simulink model "ClimaCenCfgMgr"  (R2022b) (continued)
== system ClimaCenCfgMgr == (continued)
  CarCfgForClimaCtrl_CarCfgForRemEngStrtPreCdn/1 -> ClimaCenCfgMgr_Runnable_sys/36(CarCfgForClimaCtrl_CarCfgForRemEngStrtPreCdn_read)
  CarCfgForClimaCtrl_CarCfgForRoofHatch/1 -> ClimaCenCfgMgr_Runnable_sys/37(CarCfgForClimaCtrl_CarCfgForRoofHatch_read)
  CarCfgForClimaCtrl_CarCfgForSeatHeatgCal/1 -> ClimaCenCfgMgr_Runnable_sys/38(CarCfgForClimaCtrl_CarCfgForSeatHeatgCal_read)
  CarCfgForClimaCtrl_CarCfgForSeatHeatgForRowFirst/1 -> ClimaCenCfgMgr_Runnable_sys/39(CarCfgForClimaCtrl_CarCfgForSeatHeatgForRowFirst_read)
  CarCfgForClimaCtrl_CarCfgForSeatHeatgForRowSec/1 -> ClimaCenCfgMgr_Runnable_sys/40(CarCfgForClimaCtrl_CarCfgForSeatHeatgForRowSec_read)
  CarCfgForClimaCtrl_CarCfgForSeatVentnForRowFirst/1 -> ClimaCenCfgMgr_Runnable_sys/41(CarCfgForClimaCtrl_CarCfgForSeatVentnForRowFirst_read)
  CarCfgForClimaCtrl_CarCfgForSeatVentnForRowSec/1 -> ClimaCenCfgMgr_Runnable_sys/42(CarCfgForClimaCtrl_CarCfgForSeatVentnForRowSec_read)
  CarCfgForClimaCtrl_CarCfgForSecRowVentnCenter/1 -> ClimaCenCfgMgr_Runnable_sys/43(CarCfgForClimaCtrl_CarCfgForSecRowVentnCenter_read)
  CarCfgForClimaCtrl_CarCfgForSecRowVentnSide/1 -> ClimaCenCfgMgr_Runnable_sys/44(CarCfgForClimaCtrl_CarCfgForSecRowVentnSide_read)
  CarCfgForClimaCtrl_CarCfgForSmallFlowCyc/1 -> ClimaCenCfgMgr_Runnable_sys/45(CarCfgForClimaCtrl_CarCfgForSmallFlowCyc_read)
  CarCfgForClimaCtrl_CarCfgForSmkSnsr/1 -> ClimaCenCfgMgr_Runnable_sys/46(CarCfgForClimaCtrl_CarCfgForSmkSnsr_read)
  CarCfgForClimaCtrl_CarCfgForSnsrForCmptmtT/1 -> ClimaCenCfgMgr_Runnable_sys/47(CarCfgForClimaCtrl_CarCfgForSnsrForCmptmtT_read)
  CarCfgForClimaCtrl_CarCfgForSnsrHumPrsnt/1 -> ClimaCenCfgMgr_Runnable_sys/48(CarCfgForClimaCtrl_CarCfgForSnsrHumPrsnt_read)
  CarCfgForClimaCtrl_CarCfgForStartDevice/1 -> ClimaCenCfgMgr_Runnable_sys/49(CarCfgForClimaCtrl_CarCfgForStartDevice_read)
  CarCfgForClimaCtrl_CarCfgForSteerWhlHeatg/1 -> ClimaCenCfgMgr_Runnable_sys/50(CarCfgForClimaCtrl_CarCfgForSteerWhlHeatg_read)
  CarCfgForClimaCtrl_CarCfgForSteerWhlPosn/1 -> ClimaCenCfgMgr_Runnable_sys/51(CarCfgForClimaCtrl_CarCfgForSteerWhlPosn_read)
  CarCfgForClimaCtrl_CarCfgForSunSnsr/1 -> ClimaCenCfgMgr_Runnable_sys/52(CarCfgForClimaCtrl_CarCfgForSunSnsr_read)
  CarCfgForClimaCtrl_CarCfgForTelmNodTyp/1 -> ClimaCenCfgMgr_Runnable_sys/53(CarCfgForClimaCtrl_CarCfgForTelmNodTyp_read)
  CarCfgForClimaCtrl_CarCfgForThermMngt/1 -> ClimaCenCfgMgr_Runnable_sys/54(CarCfgForClimaCtrl_CarCfgForThermMngt_read)
  CarCfgForClimaCtrl_CarCfgForVehTyp/1 -> ClimaCenCfgMgr_Runnable_sys/55(CarCfgForClimaCtrl_CarCfgForVehTyp_read)
  CarCfgForClimaCtrl_CarCfgForVgaMotorExistSts/1 -> ClimaCenCfgMgr_Runnable_sys/56(CarCfgForClimaCtrl_CarCfgForVgaMotorExistSts_read)
  CarCfgForClimaCtrl_CarCfgForWindHeatd/1 -> ClimaCenCfgMgr_Runnable_sys/57(CarCfgForClimaCtrl_CarCfgForWindHeatd_read)
  CooltVlvOutpTyp_CooltVlvOutpTyp/1 -> ClimaCenCfgMgr_Runnable_sys/58(CooltVlvOutpTyp_CooltVlvOutpTyp_read)
  HvacCfgForClimaCtrl_HvacCfgForHvacFrontBlowerType/1 -> ClimaCenCfgMgr_Runnable_sys/59(HvacCfgForClimaCtrl_HvacCfgForHvacFrontBlowerType_read)
  HvacCfgForClimaCtrl_HvacCfgForHvacMiscExistSts/1 -> ClimaCenCfgMgr_Runnable_sys/60(HvacCfgForClimaCtrl_HvacCfgForHvacMiscExistSts_read)
  HvacCfgForClimaCtrl_HvacCfgForHvacMotorExistSts/1 -> ClimaCenCfgMgr_Runnable_sys/61(HvacCfgForClimaCtrl_HvacCfgForHvacMotorExistSts_read)
  HvacCfgForClimaCtrl_HvacCfgForHvacSensorExistSts/1 -> ClimaCenCfgMgr_Runnable_sys/62(HvacCfgForClimaCtrl_HvacCfgForHvacSensorExistSts_read)
  CarCfgForClimaCtrl_CarCfgFor3RDROWSEATHEAT/1 -> ClimaCenCfgMgr_Runnable_sys/63(CarCfgForClimaCtrl_CarCfgFor3RDROWSEATHEAT_read)
  CarCfgForClimaCtrl_CarCfgFor3RDROWSEATVENTILATED/1 -> ClimaCenCfgMgr_Runnable_sys/64(CarCfgForClimaCtrl_CarCfgFor3RDROWSEATVENTILATED_read)
  CarCfgForClimaCtrl_CarCfgForBRAND/1 -> ClimaCenCfgMgr_Runnable_sys/65(CarCfgForClimaCtrl_CarCfgForBRAND_read)
  CarCfgForClimaCtrl_CarCfgForCabinOxgenSupply/1 -> ClimaCenCfgMgr_Runnable_sys/66(CarCfgForClimaCtrl_CarCfgForCabinOxgenSupply_read)
  CarCfgForClimaCtrl_CarCfgForElectricLiftingModule/1 -> ClimaCenCfgMgr_Runnable_sys/67(CarCfgForClimaCtrl_CarCfgForElectricLiftingModule_read)
  CarCfgForClimaCtrl_CarCfgForFloatingDriving/1 -> ClimaCenCfgMgr_Runnable_sys/68(CarCfgForClimaCtrl_CarCfgForFloatingDriving_read)
  CarCfgForClimaCtrl_CarCfgForInteriorViewCamera/1 -> ClimaCenCfgMgr_Runnable_sys/69(CarCfgForClimaCtrl_CarCfgForInteriorViewCamera_read)
  CarCfgForClimaCtrl_CarCfgForLifeDetection/1 -> ClimaCenCfgMgr_Runnable_sys/70(CarCfgForClimaCtrl_CarCfgForLifeDetection_read)
  CarCfgForClimaCtrl_CarCfgForMARKET/1 -> ClimaCenCfgMgr_Runnable_sys/71(CarCfgForClimaCtrl_CarCfgForMARKET_read)
  CarCfgForClimaCtrl_CarCfgForPowerSeats/1 -> ClimaCenCfgMgr_Runnable_sys/72(CarCfgForClimaCtrl_CarCfgForPowerSeats_read)
  CarCfgForClimaCtrl_CarCfgForPTCInFrontHVAC/1 -> ClimaCenCfgMgr_Runnable_sys/73(CarCfgForClimaCtrl_CarCfgForPTCInFrontHVAC_read)
  CarCfgForClimaCtrl_CarCfgForTEMPEREDSTORAGEBOX/1 -> ClimaCenCfgMgr_Runnable_sys/75(CarCfgForClimaCtrl_CarCfgForTEMPEREDSTORAGEBOX_read)
  CarCfgForClimaCtrl_CarCfgForVentLiftMotorExiSts/1 -> ClimaCenCfgMgr_Runnable_sys/76(CarCfgForClimaCtrl_CarCfgForVentLiftMotorExiSts_read)
  CarCfgForClimaCtrl_CarCfgForWadingAssistSystem/1 -> ClimaCenCfgMgr_Runnable_sys/77(CarCfgForClimaCtrl_CarCfgForWadingAssistSystem_read)
  CarCfgForClimaCtrl_CarCfgWindFollowHumanMov/1 -> ClimaCenCfgMgr_Runnable_sys/78(CarCfgForClimaCtrl_CarCfgWindFollowHumanMov_read)
  CenCfgMgrForCompile_CarCfgForAirClnrAdv/1 -> ClimaCenCfgMgr_Runnable_sys/79(CenCfgMgrForCompile_CarCfgForAirClnrAdv_read)
  CenCfgMgrForCompile_CarCfgForAutDemst/1 -> ClimaCenCfgMgr_Runnable_sys/80(CenCfgMgrForCompile_CarCfgForAutDemst_read)
  CenCfgMgrForCompile_CarCfgForCmprAcTyp3/1 -> ClimaCenCfgMgr_Runnable_sys/81(CenCfgMgrForCompile_CarCfgForCmprAcTyp3_read)
  CenCfgMgrForCompile_CarCfgForHeatrTypGly/1 -> ClimaCenCfgMgr_Runnable_sys/82(CenCfgMgrForCompile_CarCfgForHeatrTypGly_read)
  CenCfgMgrForCompile_CarCfgForSteerWhlHeatg/1 -> ClimaCenCfgMgr_Runnable_sys/83(CenCfgMgrForCompile_CarCfgForSteerWhlHeatg_read)
  HvacCfgForClimaCtrl_HvacCfgForAQSTypes/1 -> ClimaCenCfgMgr_Runnable_sys/84(HvacCfgForClimaCtrl_HvacCfgForAQSTypes_read)
  HvacCfgForClimaCtrl_HvacCfgForHvacLowSideRETXVESts/1 -> ClimaCenCfgMgr_Runnable_sys/85(HvacCfgForClimaCtrl_HvacCfgForHvacLowSideRETXVESts_read)
  HvacCfgForClimaCtrl_HvacCfgForHvacReBlowerType/1 -> ClimaCenCfgMgr_Runnable_sys/86(HvacCfgForClimaCtrl_HvacCfgForHvacReBlowerType_read)
  HvacCfgForClimaCtrl_HvacCfgForHvacReVentPstn/1 -> ClimaCenCfgMgr_Runnable_sys/87(HvacCfgForClimaCtrl_HvacCfgForHvacReVentPstn_read)
  HvacCfgForClimaCtrl_HvacCfgForHvacSnsrRCmptmtESts/1 -> ClimaCenCfgMgr_Runnable_sys/88(HvacCfgForClimaCtrl_HvacCfgForHvacSnsrRCmptmtESts_read)
  HvacCfgForClimaCtrl_HvacCfgForHvacSnsrReHexESts/1 -> ClimaCenCfgMgr_Runnable_sys/89(HvacCfgForClimaCtrl_HvacCfgForHvacSnsrReHexESts_read)
  HvacCfgReqVersionReplace_HvacCfgReqVersionReplace/1 -> ClimaCenCfgMgr_Runnable_sys/90(HvacCfgReqVersionReplace_HvacCfgReqVersionReplace_read)
  ClimaCenCfgMgr_Runnable_sys/1(ClimaCfgFor3RDROWSEATHEAT_ClimaCfgFor3RDROWSEATHEAT_write) -> ClimaCfgFor3RDROWSEATHEAT_ClimaCfgFor3RDROWSEATHEAT/1
  ClimaCenCfgMgr_Runnable_sys/2(ClimaCfgFor3RDROWSEATVENTILATED_ClimaCfgFor3RDROWSEATVENTILATED_write) -> ClimaCfgFor3RDROWSEATVENTILATED_ClimaCfgFor3RDROWSEATVENTILATED/1
  ClimaCenCfgMgr_Runnable_sys/3(ClimaCfgForAAC_ClimaCfgForAAC_write) -> ClimaCfgForAAC_ClimaCfgForAAC/1
  ClimaCenCfgMgr_Runnable_sys/4(ClimaCfgForAQSTypes_ClimaCfgForAQSTypes_write) -> ClimaCfgForAQSTypes_ClimaCfgForAQSTypes/1
  ClimaCenCfgMgr_Runnable_sys/5(ClimaCfgForAutDemst_ClimaCfgForAutDemst_write) -> ClimaCfgForAutDemst_ClimaCfgForAutDemst/1
  ClimaCenCfgMgr_Runnable_sys/6(ClimaCfgForBRAND_ClimaCfgForBRAND_write) -> ClimaCfgForBRAND_ClimaCfgForBRAND/1
  ClimaCenCfgMgr_Runnable_sys/7(ClimaCfgForCabinOxgenSupply_ClimaCfgForCabinOxgenSupply_write) -> ClimaCfgForCabinOxgenSupply_ClimaCfgForCabinOxgenSupply/1
  ClimaCenCfgMgr_Runnable_sys/8(ClimaCfgForDrvrSeatVentWthSmf2_ClimaCfgForDrvrSeatVentWthSmf2_write) -> ClimaCfgForDrvrSeatVentWthSmf2_ClimaCfgForDrvrSeatVentWthSmf2/1
  ClimaCenCfgMgr_Runnable_sys/9(ClimaCfgForElectricLiftingModule_ClimaCfgForElectricLiftingModule_write) -> ClimaCfgForElectricLiftingModule_ClimaCfgForElectricLiftingModule/1
  ClimaCenCfgMgr_Runnable_sys/10(ClimaCfgForErev1_ClimaCfgForErev1_write) -> ClimaCfgForErev1_ClimaCfgForErev1/1
  ClimaCenCfgMgr_Runnable_sys/11(ClimaCfgForFLOATINGDRVING_ClimaCfgForFLOATINGDRVING_write) -> ClimaCfgForFLOATINGDRVING_ClimaCfgForFLOATINGDRVING/1
  ClimaCenCfgMgr_Runnable_sys/12(ClimaCfgForHvacLowSideRETXVESts_ClimaCfgForHvacLowSideRETXVESts_write) -> ClimaCfgForHvacLowSideRETXVESts_ClimaCfgForHvacLowSideRETXVESts/1
  ClimaCenCfgMgr_Runnable_sys/13(ClimaCfgForHvacReBlowerType_ClimaCfgForHvacReBlowerType_write) -> ClimaCfgForHvacReBlowerType_ClimaCfgForHvacReBlowerType/1
  ClimaCenCfgMgr_Runnable_sys/14(ClimaCfgForHvacReTmpZn_ClimaCfgForHvacReTmpZn_write) -> ClimaCfgForHvacReTmpZn_ClimaCfgForHvacReTmpZn/1
  ClimaCenCfgMgr_Runnable_sys/15(ClimaCfgForHvacReVentPstn_ClimaCfgForRowSecAtFlrLeRe_write) -> ClimaCfgForHvacReVentPstn_ClimaCfgForRowSecAtFlrLeRe/1
  ClimaCenCfgMgr_Runnable_sys/16(ClimaCfgForHvacReVentPstn_ClimaCfgForRowSecAtFlrRiRe_write) -> ClimaCfgForHvacReVentPstn_ClimaCfgForRowSecAtFlrRiRe/1
  ClimaCenCfgMgr_Runnable_sys/17(ClimaCfgForHvacReVentPstn_ClimaCfgForRowSecAtVentSideTopLe_write) -> ClimaCfgForHvacReVentPstn_ClimaCfgForRowSecAtVentSideTopLe/1
  ClimaCenCfgMgr_Runnable_sys/18(ClimaCfgForHvacReVentPstn_ClimaCfgForRowSecAtVentSideTopRi_write) -> ClimaCfgForHvacReVentPstn_ClimaCfgForRowSecAtVentSideTopRi/1
  ClimaCenCfgMgr_Runnable_sys/19(ClimaCfgForHvacReVentPstn_ClimaCfgForRowThrdAtFlrLe_write) -> ClimaCfgForHvacReVentPstn_ClimaCfgForRowThrdAtFlrLe/1
  ClimaCenCfgMgr_Runnable_sys/20(ClimaCfgForHvacReVentPstn_ClimaCfgForRowThrdAtFlrRi_write) -> ClimaCfgForHvacReVentPstn_ClimaCfgForRowThrdAtFlrRi/1
  ClimaCenCfgMgr_Runnable_sys/21(ClimaCfgForHvacReVentPstn_ClimaCfgForRowThrdAtVentSideTopLe_write) -> ClimaCfgForHvacReVentPstn_ClimaCfgForRowThrdAtVentSideTopLe/1
  ClimaCenCfgMgr_Runnable_sys/22(ClimaCfgForHvacReVentPstn_ClimaCfgForRowThrdAtVentSideTopRi_write) -> ClimaCfgForHvacReVentPstn_ClimaCfgForRowThrdAtVentSideTopRi/1
  ClimaCenCfgMgr_Runnable_sys/23(ClimaCfgForHvacSnsrRCmptmtESts_ClimaCfgForHvacSnsrRCmptmtESts_write) -> ClimaCfgForHvacSnsrRCmptmtESts_ClimaCfgForHvacSnsrRCmptmtESts/1
  ClimaCenCfgMgr_Runnable_sys/24(ClimaCfgForHvacSnsrReHexESts_ClimaCfgForHvacSnsrReHexESts_write) -> ClimaCfgForHvacSnsrReHexESts_ClimaCfgForHvacSnsrReHexESts/1
  ClimaCenCfgMgr_Runnable_sys/25(ClimaCfgForHvacTyp_ClimaCfgForHvacTyp_write) -> ClimaCfgForHvacTyp_ClimaCfgForHvacTyp/1
  ClimaCenCfgMgr_Runnable_sys/26(ClimaCfgForInteriorViewCamera_ClimaCfgForInteriorViewCamera_write) -> ClimaCfgForInteriorViewCamera_ClimaCfgForInteriorViewCamera/1
  ClimaCenCfgMgr_Runnable_sys/27(ClimaCfgForLifeDetection_ClimaCfgForLifeDetection_write) -> ClimaCfgForLifeDetection_ClimaCfgForLifeDetection/1
  ClimaCenCfgMgr_Runnable_sys/28(ClimaCfgForMARKET_ClimaCfgForMARKET_write) -> ClimaCfgForMARKET_ClimaCfgForMARKET/1
  ClimaCenCfgMgr_Runnable_sys/29(ClimaCfgForPassSeatVentWthSmf2_ClimaCfgForPassSeatVentWthSmf2_write) -> ClimaCfgForPassSeatVentWthSmf2_ClimaCfgForPassSeatVentWthSmf2/1
  ClimaCenCfgMgr_Runnable_sys/30(ClimaCfgForPowerSeats_ClimaCfgForPowerSeats_write) -> ClimaCfgForPowerSeats_ClimaCfgForPowerSeats/1
  ClimaCenCfgMgr_Runnable_sys/31(ClimaCfgForPTCInFrontHVAC_ClimaCfgForPTCInFrontHVAC_write) -> ClimaCfgForPTCInFrontHVAC_ClimaCfgForPTCInFrontHVAC/1
  ClimaCenCfgMgr_Runnable_sys/32(ClimaCfgForRFKEY_ClimaCfgForRFKEY_write) -> ClimaCfgForRFKEY_ClimaCfgForRFKEY/1
  ClimaCenCfgMgr_Runnable_sys/33(ClimaCfgForTEMPEREDSTORAGEBOX_ClimaCfgForTEMPEREDSTORAGEBOX_write) -> ClimaCfgForTEMPEREDSTORAGEBOX_ClimaCfgForTEMPEREDSTORAGEBOX/1
  ClimaCenCfgMgr_Runnable_sys/34(ClimaCfgForVgaMotor1_ClimaCfgForVgaMotor1_write) -> ClimaCfgForVgaMotor1_ClimaCfgForVgaMotor1/1
  ClimaCenCfgMgr_Runnable_sys/35(ClimaCfgForVgaMotor10_ClimaCfgForVgaMotor10_write) -> ClimaCfgForVgaMotor10_ClimaCfgForVgaMotor10/1
  ClimaCenCfgMgr_Runnable_sys/36(ClimaCfgForVgaMotor11_ClimaCfgForVgaMotor11_write) -> ClimaCfgForVgaMotor11_ClimaCfgForVgaMotor11/1
  ClimaCenCfgMgr_Runnable_sys/37(ClimaCfgForVgaMotor12_ClimaCfgForVgaMotor12_write) -> ClimaCfgForVgaMotor12_ClimaCfgForVgaMotor12/1
  ClimaCenCfgMgr_Runnable_sys/38(ClimaCfgForVgaMotor13_ClimaCfgForVgaMotor13_write) -> ClimaCfgForVgaMotor13_ClimaCfgForVgaMotor13/1
  ClimaCenCfgMgr_Runnable_sys/39(ClimaCfgForVgaMotor14_ClimaCfgForVgaMotor14_write) -> ClimaCfgForVgaMotor14_ClimaCfgForVgaMotor14/1
  ClimaCenCfgMgr_Runnable_sys/40(ClimaCfgForVgaMotor15_ClimaCfgForVgaMotor15_write) -> ClimaCfgForVgaMotor15_ClimaCfgForVgaMotor15/1
  ClimaCenCfgMgr_Runnable_sys/41(ClimaCfgForVgaMotor16_ClimaCfgForVgaMotor16_write) -> ClimaCfgForVgaMotor16_ClimaCfgForVgaMotor16/1
  ClimaCenCfgMgr_Runnable_sys/42(ClimaCfgForVgaMotor17_ClimaCfgForVgaMotor17_write) -> ClimaCfgForVgaMotor17_ClimaCfgForVgaMotor17/1
  ClimaCenCfgMgr_Runnable_sys/43(ClimaCfgForVgaMotor18_ClimaCfgForVgaMotor18_write) -> ClimaCfgForVgaMotor18_ClimaCfgForVgaMotor18/1
  ClimaCenCfgMgr_Runnable_sys/44(ClimaCfgForVgaMotor19_ClimaCfgForVgaMotor19_write) -> ClimaCfgForVgaMotor19_ClimaCfgForVgaMotor19/1
  ClimaCenCfgMgr_Runnable_sys/45(ClimaCfgForVgaMotor2_ClimaCfgForVgaMotor2_write) -> ClimaCfgForVgaMotor2_ClimaCfgForVgaMotor2/1
  ClimaCenCfgMgr_Runnable_sys/46(ClimaCfgForVgaMotor20_ClimaCfgForVgaMotor20_write) -> ClimaCfgForVgaMotor20_ClimaCfgForVgaMotor20/1
  ClimaCenCfgMgr_Runnable_sys/47(ClimaCfgForVgaMotor3_ClimaCfgForVgaMotor3_write) -> ClimaCfgForVgaMotor3_ClimaCfgForVgaMotor3/1
  ClimaCenCfgMgr_Runnable_sys/48(ClimaCfgForVgaMotor4_ClimaCfgForVgaMotor4_write) -> ClimaCfgForVgaMotor4_ClimaCfgForVgaMotor4/1
  ClimaCenCfgMgr_Runnable_sys/49(ClimaCfgForVgaMotor5_ClimaCfgForVgaMotor5_write) -> ClimaCfgForVgaMotor5_ClimaCfgForVgaMotor5/1
  ClimaCenCfgMgr_Runnable_sys/50(ClimaCfgForVgaMotor6_ClimaCfgForVgaMotor6_write) -> ClimaCfgForVgaMotor6_ClimaCfgForVgaMotor6/1
  ClimaCenCfgMgr_Runnable_sys/51(ClimaCfgForVgaMotor7_ClimaCfgForVgaMotor7_write) -> ClimaCfgForVgaMotor7_ClimaCfgForVgaMotor7/1
  ClimaCenCfgMgr_Runnable_sys/52(ClimaCfgForVgaMotor8_ClimaCfgForVgaMotor8_write) -> ClimaCfgForVgaMotor8_ClimaCfgForVgaMotor8/1
  ClimaCenCfgMgr_Runnable_sys/53(ClimaCfgForVgaMotor9_ClimaCfgForVgaMotor9_write) -> ClimaCfgForVgaMotor9_ClimaCfgForVgaMotor9/1
  ClimaCenCfgMgr_Runnable_sys/54(ClimaCfgForWadingAssistSystem_ClimaCfgForWadingAssistSystem_write) -> ClimaCfgForWadingAssistSystem_ClimaCfgForWadingAssistSystem/1
  ClimaCenCfgMgr_Runnable_sys/55(ClimaCfgHvacForFrntLeTempActr_ClimaCfgHvacForFrntLeTempActr_write) -> ClimaCfgHvacForFrntLeTempActr_ClimaCfgHvacForFrntLeTempActr/1
  ClimaCenCfgMgr_Runnable_sys/56(ClimaCfgHvacForFrntRiTempActr_ClimaCfgHvacForFrntRiTempActr_write) -> ClimaCfgHvacForFrntRiTempActr_ClimaCfgHvacForFrntRiTempActr/1
  ClimaCenCfgMgr_Runnable_sys/57(ClimaCfgHvacFrntForRowSecForActrTLe_ClimaCfgHvacFrntForRowSecForActrTLe_write) -> ClimaCfgHvacFrntForRowSecForActrTLe_ClimaCfgHvacFrntForRowSecForActrTLe/1
  ClimaCenCfgMgr_Runnable_sys/58(ClimaCfgHvacFrntForRowSecForActrTRi_ClimaCfgHvacFrntForRowSecForActrTRi_write) -> ClimaCfgHvacFrntForRowSecForActrTRi_ClimaCfgHvacFrntForRowSecForActrTRi/1
  ClimaCenCfgMgr_Runnable_sys/59(ClimaCfgHvacReForRowSecForActrTLe_ClimaCfgHvacReForRowSecForActrTLe_write) -> ClimaCfgHvacReForRowSecForActrTLe_ClimaCfgHvacReForRowSecForActrTLe/1
  ClimaCenCfgMgr_Runnable_sys/60(ClimaCfgHvacReForRowSecForActrTRi_ClimaCfgHvacReForRowSecForActrTRi_write) -> ClimaCfgHvacReForRowSecForActrTRi_ClimaCfgHvacReForRowSecForActrTRi/1
  ClimaCenCfgMgr_Runnable_sys/61(ClimaCfgReqVersionReplace_ClimaCfgReqVersionReplace_write) -> ClimaCfgReqVersionReplace_ClimaCfgReqVersionReplace/1
  ClimaCenCfgMgr_Runnable_sys/62(ClimaCfgWindFollowHumanMov_ClimaCfgWindFollowHumanMov_write) -> ClimaCfgWindFollowHumanMov_ClimaCfgWindFollowHumanMov/1
  ClimaCenCfgMgr_Runnable_sys/63(ClimaCfgForActrModVrnt1_ClimaCfgForActrInd1_write) -> ClimaCfgForActrModVrnt1_ClimaCfgForActrInd1/1
  ClimaCenCfgMgr_Runnable_sys/64(ClimaCfgForActrModVrnt1_ClimaCfgForActrModVrnt1_write) -> ClimaCfgForActrModVrnt1_ClimaCfgForActrModVrnt1/1
  ClimaCenCfgMgr_Runnable_sys/65(ClimaCfgForActrModVrnt1_ClimaCfgForActrModVrntCen_write) -> ClimaCfgForActrModVrnt1_ClimaCfgForActrModVrntCen/1
  ClimaCenCfgMgr_Runnable_sys/66(ClimaCfgForActrModVrnt2_ClimaCfgForActrInd2_write) -> ClimaCfgForActrModVrnt2_ClimaCfgForActrInd2/1
  ClimaCenCfgMgr_Runnable_sys/67(ClimaCfgForActrModVrnt2_ClimaCfgForActrModVrnt2_write) -> ClimaCfgForActrModVrnt2_ClimaCfgForActrModVrnt2/1
  ClimaCenCfgMgr_Runnable_sys/68(ClimaCfgForActrRecircAndOsaCmnPrsnt1_ClimaCfgForActrRecircAndOsaCmnPrsnt1_write) -> ClimaCfgForActrRecircAndOsaCmnPrsnt1_ClimaCfgForActrRecircAndOsaCmnPrsnt1/1
  ClimaCenCfgMgr_Runnable_sys/69(ClimaCfgForAirClng1_ClimaCfgForAirClng1_write) -> ClimaCfgForAirClng1_ClimaCfgForAirClng1/1
  ClimaCenCfgMgr_Runnable_sys/70(ClimaCfgForAirClnrAdv1_ClimaCfgForAirClnrAdv1_write) -> ClimaCfgForAirClnrAdv1_ClimaCfgForAirClnrAdv1/1
  ClimaCenCfgMgr_Runnable_sys/71(ClimaCfgForAirFrag_ClimaCfgForAirFrag_write) -> ClimaCfgForAirFrag_ClimaCfgForAirFrag/1
  ClimaCenCfgMgr_Runnable_sys/72(ClimaCfgForAirMtrlSnsrAPMAPrsnt1_ClimaCfgForAirMtrlSnsrAPMAPrsnt1_write) -> ClimaCfgForAirMtrlSnsrAPMAPrsnt1_ClimaCfgForAirMtrlSnsrAPMAPrsnt1/1
  ClimaCfgForAirMtrlSnsrPrsnt1_ClimaCfgForAirMtrlSnsrPrsnt1_merge/1 -> ClimaCfgForAirMtrlSnsrPrsnt1_ClimaCfgForAirMtrlSnsrPrsnt1/1
  ClimaCenCfgMgr_Runnable_sys/74(ClimaCfgForAirQly1_ClimaCfgForAirQly1_write) -> ClimaCfgForAirQly1_ClimaCfgForAirQly1/1
  ClimaCenCfgMgr_Runnable_sys/75(ClimaCfgForAmntOfSeat1_ClimaCfgForAmntOfSeat1_write) -> ClimaCfgForAmntOfSeat1_ClimaCfgForAmntOfSeat1/1
  ClimaCenCfgMgr_Runnable_sys/76(ClimaCfgForAmntOfSeatRow1_ClimaCfgForAmntOfSeatRow1_write) -> ClimaCfgForAmntOfSeatRow1_ClimaCfgForAmntOfSeatRow1/1
  ClimaCenCfgMgr_Runnable_sys/77(ClimaCfgForBlowrInRowSecVentSidePrsnt1_ClimaCfgForBlowrInRowSecVentSidePrsnt1_write) -> ClimaCfgForBlowrInRowSecVentSidePrsnt1_ClimaCfgForBlowrInRowSecVentSidePrsnt1/1
  ClimaCenCfgMgr_Runnable_sys/78(ClimaCfgForBypVlvInHvacExtCtrl1_ClimaCfgForBypVlvInHvacExtCtrl1_write) -> ClimaCfgForBypVlvInHvacExtCtrl1_ClimaCfgForBypVlvInHvacExtCtrl1/1
  ClimaCenCfgMgr_Runnable_sys/79(ClimaCfgForBypVlvInHvacPrsnt1_ClimaCfgForBypVlvInHvacPrsnt1_write) -> ClimaCfgForBypVlvInHvacPrsnt1_ClimaCfgForBypVlvInHvacPrsnt1/1
  ClimaCenCfgMgr_Runnable_sys/80(ClimaCfgForCarTyp1_ClimaCfgForCarTyp1_write) -> ClimaCfgForCarTyp1_ClimaCfgForCarTyp1/1
  ClimaCenCfgMgr_Runnable_sys/81(ClimaCfgForCds1_ClimaCfgForCds1_write) -> ClimaCfgForCds1_ClimaCfgForCds1/1
  ClimaCenCfgMgr_Runnable_sys/82(ClimaCfgForClimaCtrlMan1_ClimaCfgForClimaCtrlMan1_write) -> ClimaCfgForClimaCtrlMan1_ClimaCfgForClimaCtrlMan1/1
  ClimaCenCfgMgr_Runnable_sys/83(ClimaCfgForClimaCtrlSwtModlPrsnt_ClimaCfgForClimaCtrlSwtModlPrsnt_write) -> ClimaCfgForClimaCtrlSwtModlPrsnt_ClimaCfgForClimaCtrlSwtModlPrsnt/1
  ClimaCenCfgMgr_Runnable_sys/84(ClimaCfgForCmptmtClng_ClimaCfgForCmptmtClng_write) -> ClimaCfgForCmptmtClng_ClimaCfgForCmptmtClng/1
  ClimaCenCfgMgr_Runnable_sys/85(ClimaCfgForCmptmtSnsr1_ClimaCfgForCmptmtSnsr1_write) -> ClimaCfgForCmptmtSnsr1_ClimaCfgForCmptmtSnsr1/1
  ClimaCenCfgMgr_Runnable_sys/86(ClimaCfgForComIfGenn2ForTelm1_ClimaCfgForComIfGenn2ForTelm1_write) -> ClimaCfgForComIfGenn2ForTelm1_ClimaCfgForComIfGenn2ForTelm1/1
  ClimaCenCfgMgr_Runnable_sys/87(ClimaCfgForCoolgPreCdn1_ClimaCfgForCoolgPreCdn1_write) -> ClimaCfgForCoolgPreCdn1_ClimaCfgForCoolgPreCdn1/1
  ClimaCenCfgMgr_Runnable_sys/88(ClimaCfgForCooltCircForClima1_ClimaCfgForCooltCircForClima1_write) -> ClimaCfgForCooltCircForClima1_ClimaCfgForCooltCircForClima1/1
  ClimaCenCfgMgr_Runnable_sys/89(ClimaCfgForCpilHeatrElecPrsnt1_ClimaCfgForCpilHeatrElecPrsnt1_write) -> ClimaCfgForCpilHeatrElecPrsnt1_ClimaCfgForCpilHeatrElecPrsnt1/1
  ClimaCenCfgMgr_Runnable_sys/90(ClimaCfgForEcuCooltVlvOutp1_ClimaCfgForEcuCooltVlvOutp1_write) -> ClimaCfgForEcuCooltVlvOutp1_ClimaCfgForEcuCooltVlvOutp1/1
  ClimaCenCfgMgr_Runnable_sys/91(ClimaCfgForEngCooltPmpElec1_ClimaCfgForEngCooltPmpElec1_write) -> ClimaCfgForEngCooltPmpElec1_ClimaCfgForEngCooltPmpElec1/1
  ClimaCenCfgMgr_Runnable_sys/92(ClimaCfgForExtVentnClimaCtrl_ClimaCfgForExtVentnClimaCtrl_write) -> ClimaCfgForExtVentnClimaCtrl_ClimaCfgForExtVentnClimaCtrl/1
  ClimaCenCfgMgr_Runnable_sys/93(ClimaCfgForFanPotiCtrld1_ClimaCfgForFanPotiCtrld1_write) -> ClimaCfgForFanPotiCtrld1_ClimaCfgForFanPotiCtrld1/1
  ClimaCfgForFlcTyp_ClimaCfgForFlcTyp_merge/1 -> ClimaCfgForFlcTyp_ClimaCfgForFlcTyp/1
  ClimaCenCfgMgr_Runnable_sys/95(ClimaCfgForFlrSnsrInHvac1_ClimaCfgForFlrSnsrInHvac1_write) -> ClimaCfgForFlrSnsrInHvac1_ClimaCfgForFlrSnsrInHvac1/1
  ClimaCenCfgMgr_Runnable_sys/96(ClimaCfgForFoldCSD_ClimaCfgForFoldCSD_write) -> ClimaCfgForFoldCSD_ClimaCfgForFoldCSD/1
  ClimaCenCfgMgr_Runnable_sys/97(ClimaCfgForForElecVentAdjmt_ClimaCfgForForElecVentAdjmt_write) -> ClimaCfgForForElecVentAdjmt_ClimaCfgForForElecVentAdjmt/1
  ClimaCenCfgMgr_Runnable_sys/98(ClimaCfgForForHeatPmp_ClimaCfgForForHeatPmp_write) -> ClimaCfgForForHeatPmp_ClimaCfgForForHeatPmp/1
  ClimaCenCfgMgr_Runnable_sys/99(ClimaCfgForForHeatPmpGly_ClimaCfgForForHeatPmp_write) -> ClimaCfgForForHeatPmpGly_ClimaCfgForForHeatPmp/1
  ClimaCfgForForLoVoltElecCfg_ClimaCfgForForLoVoltElecCfg_merge/1 -> ClimaCfgForForLoVoltElecCfg_ClimaCfgForForLoVoltElecCfg/1
  ClimaCfgForForRemEngStrtPreCdn_ClimaCfgForForRemEngStrtPreCdn_merge/1 -> ClimaCfgForForRemEngStrtPreCdn_ClimaCfgForForRemEngStrtPreCdn/1
  ClimaCenCfgMgr_Runnable_sys/102(ClimaCfgForFuHeatrFuLine1_ClimaCfgForFuHeatrFuLine1_write) -> ClimaCfgForFuHeatrFuLine1_ClimaCfgForFuHeatrFuLine1/1
  ClimaCfgForFuHeatrTyp1_ClimaCfgForFuHeatrTyp1_merge/1 -> ClimaCfgForFuHeatrTyp1_ClimaCfgForFuHeatrTyp1/1
  ClimaCenCfgMgr_Runnable_sys/104(ClimaCfgForGlassTyp1_ClimaCfgForGlassTyp1_write) -> ClimaCfgForGlassTyp1_ClimaCfgForGlassTyp1/1
  ClimaCenCfgMgr_Runnable_sys/105(ClimaCfgForHeadRadar_ClimaCfgForHeadRadar_write) -> ClimaCfgForHeadRadar_ClimaCfgForHeadRadar/1
  ClimaCenCfgMgr_Runnable_sys/106(ClimaCfgForHeatgPreCdnAvl1_ClimaCfgForHeatgPreCdnAvl1_write) -> ClimaCfgForHeatgPreCdnAvl1_ClimaCfgForHeatgPreCdnAvl1/1
  ClimaCfgForHeatrTyp1_ClimaCfgForHeatrTyp1_merge/1 -> ClimaCfgForHeatrTyp1_ClimaCfgForHeatrTyp1/1
  ClimaCfgForHvacFilVrnt1_ClimaCfgForHvacFilVrnt1_merge/1 -> ClimaCfgForHvacFilVrnt1_ClimaCfgForHvacFilVrnt1/1
  ClimaCenCfgMgr_Runnable_sys/109(ClimaCfgForHvacFrontBlowerType_ClimaCfgForHvacFrontBlowerType_write) -> ClimaCfgForHvacFrontBlowerType_ClimaCfgForHvacFrontBlowerType/1
  ClimaCenCfgMgr_Runnable_sys/110(ClimaCfgForHvacHeatrElecFrntPrsnt1_ClimaCfgForHvacHeatrElecFrntPrsnt1_write) -> ClimaCfgForHvacHeatrElecFrntPrsnt1_ClimaCfgForHvacHeatrElecFrntPrsnt1/1
  ClimaCenCfgMgr_Runnable_sys/111(ClimaCfgForHvacHeatrSnsrTPrsnt1_ClimaCfgForHvacHeatrSnsrTPrsnt1_write) -> ClimaCfgForHvacHeatrSnsrTPrsnt1_ClimaCfgForHvacHeatrSnsrTPrsnt1/1
  ClimaCenCfgMgr_Runnable_sys/112(ClimaCfgForHvacManWithPartRecirc2_ClimaCfgForHvacManWithPartRecirc2_write) -> ClimaCfgForHvacManWithPartRecirc2_ClimaCfgForHvacManWithPartRecirc2/1
  ClimaCenCfgMgr_Runnable_sys/113(ClimaCfgForHvacMiscExistSts_ClimaCfgForHvacMiscExistSts_write) -> ClimaCfgForHvacMiscExistSts_ClimaCfgForHvacMiscExistSts/1
  ClimaCenCfgMgr_Runnable_sys/114(ClimaCfgForHvacRePrsnt1_ClimaCfgForHvacRePrsnt1_write) -> ClimaCfgForHvacRePrsnt1_ClimaCfgForHvacRePrsnt1/1
  ClimaCenCfgMgr_Runnable_sys/115(ClimaCfgForHvacReTyp1_ClimaCfgForHvacReTyp1_write) -> ClimaCfgForHvacReTyp1_ClimaCfgForHvacReTyp1/1
  ClimaCenCfgMgr_Runnable_sys/116(ClimaCfgForHvacSnsrCfg1_ClimaCfgForHvacSnsrCfg1_write) -> ClimaCfgForHvacSnsrCfg1_ClimaCfgForHvacSnsrCfg1/1
  ClimaCfgForHvHeatrTyp1_ClimaCfgForHvHeatrTyp1_merge/1 -> ClimaCfgForHvHeatrTyp1_ClimaCfgForHvHeatrTyp1/1
  ClimaCfgForNavSysandIntnmap_ClimaCfgForNavSysandIntnmap_merge/1 -> ClimaCfgForNavSysandIntnmap_ClimaCfgForNavSysandIntnmap/1
  ClimaCenCfgMgr_Runnable_sys/119(ClimaCfgForNrOfAirDistbnSetg1_ClimaCfgForNrOfAirDistbnSetg1_write) -> ClimaCfgForNrOfAirDistbnSetg1_ClimaCfgForNrOfAirDistbnSetg1/1
  ClimaCenCfgMgr_Runnable_sys/120(ClimaCfgForNrOfDoor1_ClimaCfgForNrOfDoor1_write) -> ClimaCfgForNrOfDoor1_ClimaCfgForNrOfDoor1/1
  ClimaCenCfgMgr_Runnable_sys/121(ClimaCfgForNrOfFanLvlSetg1_ClimaCfgForNrOfFanLvlSetg1_write) -> ClimaCfgForNrOfFanLvlSetg1_ClimaCfgForNrOfFanLvlSetg1/1
  ClimaCenCfgMgr_Runnable_sys/122(ClimaCfgForNrOfTSetg1_ClimaCfgForNrOfTSetg1_write) -> ClimaCfgForNrOfTSetg1_ClimaCfgForNrOfTSetg1/1
  ClimaCenCfgMgr_Runnable_sys/123(ClimaCfgForPostCdnAvl1_ClimaCfgForPostCdnAvl1_write) -> ClimaCfgForPostCdnAvl1_ClimaCfgForPostCdnAvl1/1
  ClimaCenCfgMgr_Runnable_sys/124(ClimaCfgForPreClng1_ClimaCfgForPreClng1_write) -> ClimaCfgForPreClng1_ClimaCfgForPreClng1/1
  ClimaCenCfgMgr_Runnable_sys/125(ClimaCfgForPrkgCDSNotAvl_ClimaCfgForPrkgCDSNotAvl_write) -> ClimaCfgForPrkgCDSNotAvl_ClimaCfgForPrkgCDSNotAvl/1
  ClimaCfgForPrpsnTyp_ClimaCfgForPrpsnTyp_merge/1 -> ClimaCfgForPrpsnTyp_ClimaCfgForPrpsnTyp/1
  ClimaCfgForRoofHatch_ClimaCfgForRoofHatch_merge/1 -> ClimaCfgForRoofHatch_ClimaCfgForRoofHatch/1
  ClimaCenCfgMgr_Runnable_sys/128(ClimaCfgForRowSecVentCentrVrnt1_ClimaCfgForRowSecVentCentrVrnt1_write) -> ClimaCfgForRowSecVentCentrVrnt1_ClimaCfgForRowSecVentCentrVrnt1/1
  ClimaCenCfgMgr_Runnable_sys/129(ClimaCfgForRowSecVentSidePrsnt1_ClimaCfgForRowSecVentSidePrsnt1_write) -> ClimaCfgForRowSecVentSidePrsnt1_ClimaCfgForRowSecVentSidePrsnt1/1
  ClimaCenCfgMgr_Runnable_sys/130(ClimaCfgForSeatHeatgCal1_ClimaCfgForSeatHeatgCal1_write) -> ClimaCfgForSeatHeatgCal1_ClimaCfgForSeatHeatgCal1/1
  ClimaCenCfgMgr_Runnable_sys/131(ClimaCfgForSeatHeatgForRowFirstPrsnt1_ClimaCfgForSeatHeatgForRowFirstPrsnt1_write) -> ClimaCfgForSeatHeatgForRowFirstPrsnt1_ClimaCfgForSeatHeatgForRowFirstPrsnt1/1
  ClimaCfgForSeatHeatgForRowSec_ClimaCfgForSeatHeatgForRowSec_merge/1 -> ClimaCfgForSeatHeatgForRowSec_ClimaCfgForSeatHeatgForRowSec/1
  ClimaCenCfgMgr_Runnable_sys/133(ClimaCfgForSeatHeatrElmDualPrsnt1_ClimaCfgForSeatHeatrElmDualPrsnt1_write) -> ClimaCfgForSeatHeatrElmDualPrsnt1_ClimaCfgForSeatHeatrElmDualPrsnt1/1
  ClimaCenCfgMgr_Runnable_sys/134(ClimaCfgForSeatVentnForRowFirst_ClimaCfgForSeatVentnForRowFirst_write) -> ClimaCfgForSeatVentnForRowFirst_ClimaCfgForSeatVentnForRowFirst/1
  ClimaCfgForSeatVentnForRowSec_ClimaCfgForSeatVentnForRowSec_merge/1 -> ClimaCfgForSeatVentnForRowSec_ClimaCfgForSeatVentnForRowSec/1
  ClimaCenCfgMgr_Runnable_sys/136(ClimaCfgForSmkSnsrPrsnt1_ClimaCfgForSmkSnsrPrsnt1_write) -> ClimaCfgForSmkSnsrPrsnt1_ClimaCfgForSmkSnsrPrsnt1/1
  ClimaCenCfgMgr_Runnable_sys/137(ClimaCfgForSnsrHumPrsnt1_ClimaCfgForSnsrHumPrsnt1_write) -> ClimaCfgForSnsrHumPrsnt1_ClimaCfgForSnsrHumPrsnt1/1
  ClimaCenCfgMgr_Runnable_sys/138(ClimaCfgForSnsrPrsntAtRowFirstAtFlrLe_ClimaCfgForSnsrPrsntAtRowFirstAtFlrLe_write) -> ClimaCfgForSnsrPrsntAtRowFirstAtFlrLe_ClimaCfgForSnsrPrsntAtRowFirstAtFlrLe/1
  ClimaCenCfgMgr_Runnable_sys/139(ClimaCfgForSnsrPrsntAtRowFirstAtFlrRi_ClimaCfgForSnsrPrsntAtRowFirstAtFlrRi_write) -> ClimaCfgForSnsrPrsntAtRowFirstAtFlrRi_ClimaCfgForSnsrPrsntAtRowFirstAtFlrRi/1
  ClimaCenCfgMgr_Runnable_sys/140(ClimaCfgForSnsrPrsntAtRowFirstAtVentSideLe_ClimaCfgForSnsrPrsntAtRowFirstAtVentSideLe_write) -> ClimaCfgForSnsrPrsntAtRowFirstAtVentSideLe_ClimaCfgForSnsrPrsntAtRowFirstAtVentSideLe/1
  ClimaCenCfgMgr_Runnable_sys/141(ClimaCfgForSnsrPrsntAtRowFirstAtVentSideRi_ClimaCfgForSnsrPrsntAtRowFirstAtVentSideRi_write) -> ClimaCfgForSnsrPrsntAtRowFirstAtVentSideRi_ClimaCfgForSnsrPrsntAtRowFirstAtVentSideRi/1
  ClimaCenCfgMgr_Runnable_sys/142(ClimaCfgForSnsrPrsntAtRowSecAtFlrLe_ClimaCfgForSnsrPrsntAtRowSecAtFlrLe_write) -> ClimaCfgForSnsrPrsntAtRowSecAtFlrLe_ClimaCfgForSnsrPrsntAtRowSecAtFlrLe/1
  ClimaCenCfgMgr_Runnable_sys/143(ClimaCfgForSnsrPrsntAtRowSecAtFlrRi_ClimaCfgForSnsrPrsntAtRowSecAtFlrRi_write) -> ClimaCfgForSnsrPrsntAtRowSecAtFlrRi_ClimaCfgForSnsrPrsntAtRowSecAtFlrRi/1
  ClimaCenCfgMgr_Runnable_sys/144(ClimaCfgForSnsrPrsntAtRowSecAtVentLe_ClimaCfgForSnsrPrsntAtRowSecAtVentLe_write) -> ClimaCfgForSnsrPrsntAtRowSecAtVentLe_ClimaCfgForSnsrPrsntAtRowSecAtVentLe/1
  ClimaCenCfgMgr_Runnable_sys/145(ClimaCfgForSnsrPrsntAtRowSecAtVentSideLe_ClimaCfgForSnsrPrsntAtRowSecAtVentSideLe_write) -> ClimaCfgForSnsrPrsntAtRowSecAtVentSideLe_ClimaCfgForSnsrPrsntAtRowSecAtVentSideLe/1
  ClimaCfgForStatrtDevice_ClimaCfgForStartDevice_merge/1 -> ClimaCfgForStatrtDevice_ClimaCfgForStartDevice/1
  ClimaCenCfgMgr_Runnable_sys/147(ClimaCfgForSteerWhlHeatgPrsnt1_ClimaCfgForSteerWhlHeatgPrsnt1_write) -> ClimaCfgForSteerWhlHeatgPrsnt1_ClimaCfgForSteerWhlHeatgPrsnt1/1
  ClimaCenCfgMgr_Runnable_sys/148(ClimaCfgForSteerWhlLeSide1_ClimaCfgForSteerWhlLeSide1_write) -> ClimaCfgForSteerWhlLeSide1_ClimaCfgForSteerWhlLeSide1/1
  ClimaCenCfgMgr_Runnable_sys/149(ClimaCfgForStrtStp_ClimaCfgForStrtStp_write) -> ClimaCfgForStrtStp_ClimaCfgForStrtStp/1
  ClimaCfgForSunSnsr1_ClimaCfgForSunSnsr1_merge/1 -> ClimaCfgForSunSnsr1_ClimaCfgForSunSnsr1/1
  ClimaCenCfgMgr_Runnable_sys/151(ClimaCfgForThermMgrPrsnt1_ClimaCfgForThermMgrPrsnt1_write) -> ClimaCfgForThermMgrPrsnt1_ClimaCfgForThermMgrPrsnt1/1
  ClimaCenCfgMgr_Runnable_sys/152(ClimaCfgForVehElec1_ClimaCfgForVehElec1_write) -> ClimaCfgForVehElec1_ClimaCfgForVehElec1/1
  ClimaCenCfgMgr_Runnable_sys/153(ClimaCfgForVehHev1_ClimaCfgForVehHev1_write) -> ClimaCfgForVehHev1_ClimaCfgForVehHev1/1
  ClimaCenCfgMgr_Runnable_sys/154(ClimaCfgForVehHyb1_ClimaCfgForVehHyb1_write) -> ClimaCfgForVehHyb1_ClimaCfgForVehHyb1/1
  ClimaCenCfgMgr_Runnable_sys/155(ClimaCfgForVehIce1_ClimaCfgForVehIce1_write) -> ClimaCfgForVehIce1_ClimaCfgForVehIce1/1
  ClimaCenCfgMgr_Runnable_sys/156(ClimaCfgForVehTyp_ClimaCfgForVehTyp_write) -> ClimaCfgForVehTyp_ClimaCfgForVehTyp/1
  ClimaCenCfgMgr_Runnable_sys/157(ClimaCfgForVgaMotorPrsntAtRowFirstLeMid_ClimaCfgForVgaMotorPrsntAtRowFirstLeMid_write) -> ClimaCfgForVgaMotorPrsntAtRowFirstLeMid_ClimaCfgForVgaMotorPrsntAtRowFirstLeMid/1
  ClimaCenCfgMgr_Runnable_sys/158(ClimaCfgForVgaMotorPrsntAtRowFirstRiMid_ClimaCfgForVgaMotorPrsntAtRowFirstRiMid_write) -> ClimaCfgForVgaMotorPrsntAtRowFirstRiMid_ClimaCfgForVgaMotorPrsntAtRowFirstRiMid/1
  ClimaCenCfgMgr_Runnable_sys/159(ClimaCfgForVgaMotorPrsntAtRowFirstSideLe_ClimaCfgForVgaMotorPrsntAtRowFirstSideLe_write) -> ClimaCfgForVgaMotorPrsntAtRowFirstSideLe_ClimaCfgForVgaMotorPrsntAtRowFirstSideLe/1
  ClimaCenCfgMgr_Runnable_sys/160(ClimaCfgForVgaMotorPrsntAtRowFirstSideRi_ClimaCfgForVgaMotorPrsntAtRowFirstSideRi_write) -> ClimaCfgForVgaMotorPrsntAtRowFirstSideRi_ClimaCfgForVgaMotorPrsntAtRowFirstSideRi/1
  ClimaCenCfgMgr_Runnable_sys/161(ClimaCfgForVgaMotorPrsntAtRowSecSideLe_ClimaCfgForVgaMotorPrsntAtRowSecSideLe_write) -> ClimaCfgForVgaMotorPrsntAtRowSecSideLe_ClimaCfgForVgaMotorPrsntAtRowSecSideLe/1
  ClimaCenCfgMgr_Runnable_sys/162(ClimaCfgForVgaMotorPrsntAtRowSecSideRi_ClimaCfgForVgaMotorPrsntAtRowSecSideRi_write) -> ClimaCfgForVgaMotorPrsntAtRowSecSideRi_ClimaCfgForVgaMotorPrsntAtRowSecSideRi/1
  ClimaCenCfgMgr_Runnable_sys/163(ClimaCfgForVrntOfVehSysElec1_ClimaCfgForVrntOfVehSysElec1_write) -> ClimaCfgForVrntOfVehSysElec1_ClimaCfgForVrntOfVehSysElec1/1
  ClimaCenCfgMgr_Runnable_sys/164(ClimaCfgForWindHeatrPrsnt1_ClimaCfgForWindHeatrPrsnt1_write) -> ClimaCfgForWindHeatrPrsnt1_ClimaCfgForWindHeatrPrsnt1/1
  ClimaCenCfgMgr_Runnable_sys/165(ClimaCfgForWtrPmpAuxPrsnt1_ClimaCfgForWtrPmpAuxPrsnt1_write) -> ClimaCfgForWtrPmpAuxPrsnt1_ClimaCfgForWtrPmpAuxPrsnt1/1
  ClimaCenCfgMgr_Runnable_sys/150(ClimaCfgForSunSnsr1_ClimaCfgForSunSnsr1_write) -> [ClimaCfgForSunSnsr1_ClimaCfgForSunSnsr1_merge_Tag]
  [ClimaCfgForSunSnsr1_ClimaCfgForSunSnsr1_merge_Tag] -> ClimaCfgForSunSnsr1_ClimaCfgForSunSnsr1_merge/2
  ClimaCenCfgMgr_Init/15(ClimaCfgForSunSnsr1_ClimaCfgForSunSnsr1_write) -> [ClimaCfgForSunSnsr1_ClimaCfgForSunSnsr1_merge_Tag1]
  [ClimaCfgForSunSnsr1_ClimaCfgForSunSnsr1_merge_Tag1] -> ClimaCfgForSunSnsr1_ClimaCfgForSunSnsr1_merge/1
  ClimaCenCfgMgr_Runnable_sys/146(ClimaCfgForStatrtDevice_ClimaCfgForStartDevice_write) -> [ClimaCfgForStatrtDevice_ClimaCfgForStartDevice_merge_Tag]
  [ClimaCfgForStatrtDevice_ClimaCfgForStartDevice_merge_Tag] -> ClimaCfgForStatrtDevice_ClimaCfgForStartDevice_merge/2
  ClimaCenCfgMgr_Init/14(ClimaCfgForStatrtDevice_ClimaCfgForStartDevice_write) -> [ClimaCfgForStatrtDevice_ClimaCfgForStartDevice_merge_Tag1]
  [ClimaCfgForStatrtDevice_ClimaCfgForStartDevice_merge_Tag1] -> ClimaCfgForStatrtDevice_ClimaCfgForStartDevice_merge/1
  ClimaCenCfgMgr_Runnable_sys/135(ClimaCfgForSeatVentnForRowSec_ClimaCfgForSeatVentnForRowSec_write) -> [ClimaCfgForSeatVentnForRowSec_ClimaCfgForSeatV_d54ca5105f54774a]
  [ClimaCfgForSeatVentnForRowSec_ClimaCfgForSeatV_d54ca5105f54774a] -> ClimaCfgForSeatVentnForRowSec_ClimaCfgForSeatVentnForRowSec_merge/2
  ClimaCenCfgMgr_Init/13(ClimaCfgForSeatVentnForRowSec_ClimaCfgForSeatVentnForRowSec_write) -> [ClimaCfgForSeatVentnForRowSec_ClimaCfgForSeatV_d4248728e415f96c]
  [ClimaCfgForSeatVentnForRowSec_ClimaCfgForSeatV_d4248728e415f96c] -> ClimaCfgForSeatVentnForRowSec_ClimaCfgForSeatVentnForRowSec_merge/1
  ClimaCenCfgMgr_Runnable_sys/132(ClimaCfgForSeatHeatgForRowSec_ClimaCfgForSeatHeatgForRowSec_write) -> [ClimaCfgForSeatHeatgForRowSec_ClimaCfgForSeatH_a4011727af8cab0b]
  [ClimaCfgForSeatHeatgForRowSec_ClimaCfgForSeatH_a4011727af8cab0b] -> ClimaCfgForSeatHeatgForRowSec_ClimaCfgForSeatHeatgForRowSec_merge/2
  ClimaCenCfgMgr_Init/12(ClimaCfgForSeatHeatgForRowSec_ClimaCfgForSeatHeatgForRowSec_write) -> [ClimaCfgForSeatHeatgForRowSec_ClimaCfgForSeatH_933b53af3d570d7f]
  [ClimaCfgForSeatHeatgForRowSec_ClimaCfgForSeatH_933b53af3d570d7f] -> ClimaCfgForSeatHeatgForRowSec_ClimaCfgForSeatHeatgForRowSec_merge/1
  ClimaCenCfgMgr_Runnable_sys/127(ClimaCfgForRoofHatch_ClimaCfgForRoofHatch_write) -> [ClimaCfgForRoofHatch_ClimaCfgForRoofHatch_merge_Tag]
  [ClimaCfgForRoofHatch_ClimaCfgForRoofHatch_merge_Tag] -> ClimaCfgForRoofHatch_ClimaCfgForRoofHatch_merge/2
  ClimaCenCfgMgr_Init/11(ClimaCfgForRoofHatch_ClimaCfgForRoofHatch_write) -> [ClimaCfgForRoofHatch_ClimaCfgForRoofHatch_merge_Tag1]
  [ClimaCfgForRoofHatch_ClimaCfgForRoofHatch_merge_Tag1] -> ClimaCfgForRoofHatch_ClimaCfgForRoofHatch_merge/1
  ClimaCenCfgMgr_Runnable_sys/126(ClimaCfgForPrpsnTyp_ClimaCfgForPrpsnTyp_write) -> [ClimaCfgForPrpsnTyp_ClimaCfgForPrpsnTyp_merge_Tag]
  [ClimaCfgForPrpsnTyp_ClimaCfgForPrpsnTyp_merge_Tag] -> ClimaCfgForPrpsnTyp_ClimaCfgForPrpsnTyp_merge/2
  ClimaCenCfgMgr_Init/10(ClimaCfgForPrpsnTyp_ClimaCfgForPrpsnTyp_write) -> [ClimaCfgForPrpsnTyp_ClimaCfgForPrpsnTyp_merge_Tag1]
  [ClimaCfgForPrpsnTyp_ClimaCfgForPrpsnTyp_merge_Tag1] -> ClimaCfgForPrpsnTyp_ClimaCfgForPrpsnTyp_merge/1
  ClimaCenCfgMgr_Runnable_sys/118(ClimaCfgForNavSysandIntnmap_ClimaCfgForNavSysandIntnmap_write) -> [ClimaCfgForNavSysandIntnmap_ClimaCfgForNavSysa_5592954702dfd3c1]
  [ClimaCfgForNavSysandIntnmap_ClimaCfgForNavSysa_5592954702dfd3c1] -> ClimaCfgForNavSysandIntnmap_ClimaCfgForNavSysandIntnmap_merge/2
  ClimaCenCfgMgr_Init/9(ClimaCfgForNavSysandIntnmap_ClimaCfgForNavSysandIntnmap_write) -> [ClimaCfgForNavSysandIntnmap_ClimaCfgForNavSysa_c2513078f026df2f]
  [ClimaCfgForNavSysandIntnmap_ClimaCfgForNavSysa_c2513078f026df2f] -> ClimaCfgForNavSysandIntnmap_ClimaCfgForNavSysandIntnmap_merge/1
  ClimaCenCfgMgr_Runnable_sys/117(ClimaCfgForHvHeatrTyp1_ClimaCfgForHvHeatrTyp1_write) -> [ClimaCfgForHvHeatrTyp1_ClimaCfgForHvHeatrTyp1_merge_Tag]
  [ClimaCfgForHvHeatrTyp1_ClimaCfgForHvHeatrTyp1_merge_Tag] -> ClimaCfgForHvHeatrTyp1_ClimaCfgForHvHeatrTyp1_merge/2
  ClimaCenCfgMgr_Init/8(ClimaCfgForHvHeatrTyp1_ClimaCfgForHvHeatrTyp1_write) -> [ClimaCfgForHvHeatrTyp1_ClimaCfgForHvHeatrTyp1_merge_Tag1]
  [ClimaCfgForHvHeatrTyp1_ClimaCfgForHvHeatrTyp1_merge_Tag1] -> ClimaCfgForHvHeatrTyp1_ClimaCfgForHvHeatrTyp1_merge/1
  ClimaCenCfgMgr_Runnable_sys/108(ClimaCfgForHvacFilVrnt1_ClimaCfgForHvacFilVrnt1_write) -> [ClimaCfgForHvacFilVrnt1_ClimaCfgForHvacFilVrnt1_merge_Tag]
  [ClimaCfgForHvacFilVrnt1_ClimaCfgForHvacFilVrnt1_merge_Tag] -> ClimaCfgForHvacFilVrnt1_ClimaCfgForHvacFilVrnt1_merge/2
  ClimaCenCfgMgr_Init/7(ClimaCfgForHvacFilVrnt1_ClimaCfgForHvacFilVrnt1_write) -> [ClimaCfgForHvacFilVrnt1_ClimaCfgForHvacFilVrnt1_merge_Tag1]
  [ClimaCfgForHvacFilVrnt1_ClimaCfgForHvacFilVrnt1_merge_Tag1] -> ClimaCfgForHvacFilVrnt1_ClimaCfgForHvacFilVrnt1_merge/1
  ClimaCenCfgMgr_Runnable_sys/107(ClimaCfgForHeatrTyp1_ClimaCfgForHeatrTyp1_write) -> [ClimaCfgForHeatrTyp1_ClimaCfgForHeatrTyp1_merge_Tag]
  [ClimaCfgForHeatrTyp1_ClimaCfgForHeatrTyp1_merge_Tag] -> ClimaCfgForHeatrTyp1_ClimaCfgForHeatrTyp1_merge/2
  ClimaCenCfgMgr_Init/6(ClimaCfgForHeatrTyp1_ClimaCfgForHeatrTyp1_write) -> [ClimaCfgForHeatrTyp1_ClimaCfgForHeatrTyp1_merge_Tag1]
  [ClimaCfgForHeatrTyp1_ClimaCfgForHeatrTyp1_merge_Tag1] -> ClimaCfgForHeatrTyp1_ClimaCfgForHeatrTyp1_merge/1
  ClimaCenCfgMgr_Runnable_sys/103(ClimaCfgForFuHeatrTyp1_ClimaCfgForFuHeatrTyp1_write) -> [ClimaCfgForFuHeatrTyp1_ClimaCfgForFuHeatrTyp1_merge_Tag]
  [ClimaCfgForFuHeatrTyp1_ClimaCfgForFuHeatrTyp1_merge_Tag] -> ClimaCfgForFuHeatrTyp1_ClimaCfgForFuHeatrTyp1_merge/2
  ClimaCenCfgMgr_Init/5(ClimaCfgForFuHeatrTyp1_ClimaCfgForFuHeatrTyp1_write) -> [ClimaCfgForFuHeatrTyp1_ClimaCfgForFuHeatrTyp1_merge_Tag1]
  [ClimaCfgForFuHeatrTyp1_ClimaCfgForFuHeatrTyp1_merge_Tag1] -> ClimaCfgForFuHeatrTyp1_ClimaCfgForFuHeatrTyp1_merge/1
  ClimaCenCfgMgr_Runnable_sys/101(ClimaCfgForForRemEngStrtPreCdn_ClimaCfgForForRemEngStrtPreCdn_write) -> [ClimaCfgForForRemEngStrtPreCdn_ClimaCfgForForR_6967ef93a184edcf]
  [ClimaCfgForForRemEngStrtPreCdn_ClimaCfgForForR_6967ef93a184edcf] -> ClimaCfgForForRemEngStrtPreCdn_ClimaCfgForForRemEngStrtPreCdn_merge/2
  ClimaCenCfgMgr_Init/4(ClimaCfgForForRemEngStrtPreCdn_ClimaCfgForForRemEngStrtPreCdn_write) -> [ClimaCfgForForRemEngStrtPreCdn_ClimaCfgForForR_99c3680963bc867d]
  [ClimaCfgForForRemEngStrtPreCdn_ClimaCfgForForR_99c3680963bc867d] -> ClimaCfgForForRemEngStrtPreCdn_ClimaCfgForForRemEngStrtPreCdn_merge/1
  ClimaCenCfgMgr_Runnable_sys/100(ClimaCfgForForLoVoltElecCfg_ClimaCfgForForLoVoltElecCfg_write) -> [ClimaCfgForForLoVoltElecCfg_ClimaCfgForForLoVo_ea687ad20b701f29]
  [ClimaCfgForForLoVoltElecCfg_ClimaCfgForForLoVo_ea687ad20b701f29] -> ClimaCfgForForLoVoltElecCfg_ClimaCfgForForLoVoltElecCfg_merge/2
  ClimaCenCfgMgr_Init/3(ClimaCfgForForLoVoltElecCfg_ClimaCfgForForLoVoltElecCfg_write) -> [ClimaCfgForForLoVoltElecCfg_ClimaCfgForForLoVo_aece14e4c22f85b8]
  [ClimaCfgForForLoVoltElecCfg_ClimaCfgForForLoVo_aece14e4c22f85b8] -> ClimaCfgForForLoVoltElecCfg_ClimaCfgForForLoVoltElecCfg_merge/1
  ClimaCenCfgMgr_Runnable_sys/94(ClimaCfgForFlcTyp_ClimaCfgForFlcTyp_write) -> [ClimaCfgForFlcTyp_ClimaCfgForFlcTyp_merge_Tag]
  [ClimaCfgForFlcTyp_ClimaCfgForFlcTyp_merge_Tag] -> ClimaCfgForFlcTyp_ClimaCfgForFlcTyp_merge/2
  ClimaCenCfgMgr_Init/2(ClimaCfgForFlcTyp_ClimaCfgForFlcTyp_write) -> [ClimaCfgForFlcTyp_ClimaCfgForFlcTyp_merge_Tag1]
  [ClimaCfgForFlcTyp_ClimaCfgForFlcTyp_merge_Tag1] -> ClimaCfgForFlcTyp_ClimaCfgForFlcTyp_merge/1
  ClimaCenCfgMgr_Runnable_sys/73(ClimaCfgForAirMtrlSnsrPrsnt1_ClimaCfgForAirMtrlSnsrPrsnt1_write) -> [ClimaCfgForAirMtrlSnsrPrsnt1_ClimaCfgForAirMtr_a2e1e1c41c52e420]
  [ClimaCfgForAirMtrlSnsrPrsnt1_ClimaCfgForAirMtr_a2e1e1c41c52e420] -> ClimaCfgForAirMtrlSnsrPrsnt1_ClimaCfgForAirMtrlSnsrPrsnt1_merge/2
  ClimaCenCfgMgr_Init/1(ClimaCfgForAirMtrlSnsrPrsnt1_ClimaCfgForAirMtrlSnsrPrsnt1_write) -> [ClimaCfgForAirMtrlSnsrPrsnt1_ClimaCfgForAirMtr_eb9cebdc1344b2cb]
  [ClimaCfgForAirMtrlSnsrPrsnt1_ClimaCfgForAirMtr_eb9cebdc1344b2cb] -> ClimaCfgForAirMtrlSnsrPrsnt1_ClimaCfgForAirMtrlSnsrPrsnt1_merge/1
  CarCfgForClimaCtrl_CarCfgForRFKEY/1 -> [CarCfgForClimaCtrl_CarCfgForRFKEY_Tag]
  [CarCfgForClimaCtrl_CarCfgForRFKEY_Tag] -> ClimaCenCfgMgr_Init/1(CarCfgForClimaCtrl_CarCfgForRFKEY_read)
  [CarCfgForClimaCtrl_CarCfgForRFKEY_Tag] -> ClimaCenCfgMgr_Runnable_sys/74(CarCfgForClimaCtrl_CarCfgForRFKEY_read)
  ClimaCenCfgMgr_Runnable/1 -> ClimaCenCfgMgr_Runnable_sys/Trigger
